simulink model "ospfb_2x_64kc_8i_16o"  (R2021a)
settings: Solver=VariableStepAuto  StartTime=0.0  StopTime=2304  RelTol=1e-3
undefined symbols (not defined in the file or in its folder): pfb_coeff_gen_calc
libraries: xbsIndex_r4 x37193, casper_library_misc x226, casper_library_delays x184
(part 195 of 313)

== system ospfb_2x_64kc_8i_16o/fft2/fft_direct/butterfly3_4/twiddle/coeff_gen/cosin ==
  theta : Inport
  misci : Inport  Port=2
  Constant4 : xbsIndex_r4/Constant  const=0  gui_display_data_type=Fixed-point  arith_type=Unsigned  n_bits=1  bin_pt=0  preci_type=Single  exp_width=8  frac_width=24  explicit_period=on  period=1  equ=P=C  opselect=C  inp2=PCIN>>17  opr=+  inp1=P  carry=CIN  dbl_ovrd=off  block_type=constant
  Constant5 : xbsIndex_r4/Constant  const=0  gui_display_data_type=Fixed-point  arith_type=Unsigned  n_bits=1  bin_pt=0  preci_type=Single  exp_width=8  frac_width=24  explicit_period=on  period=1  equ=P=C  opselect=C  inp2=PCIN>>17  opr=+  inp1=P  carry=CIN  dbl_ovrd=off  block_type=constant
  Delay : xbsIndex_r4/Delay  rst=off  infoeditControl=Selection of Reset will increase slice count due to use of real FFs and instead of SRLs  en=off  latency=bram_latency  reg_retiming=on  dbl_ovrd=off  block_type=delay
  Delay10 : xbsIndex_r4/Delay  rst=off  infoeditControl=Selection of Reset will increase slice count due to use of real FFs and instead of SRLs  en=off  latency=bram_latency  reg_retiming=on  dbl_ovrd=off  block_type=delay
  Delay8 : xbsIndex_r4/Delay  rst=off  infoeditControl=Selection of Reset will increase slice count due to use of real FFs and instead of SRLs  en=off  latency=bram_latency  reg_retiming=on  dbl_ovrd=off  block_type=delay
  ROM : xbsIndex_r4/ROM  depth=2^(12)  initVector=[31744539665 31743228933 31741918202 31740607470 31739296738 31738248151 31736937419 31735626688 31734315956 31733005224 31731956637 31730645905 31729335174 31728024442 31726713710 31725402979 31724354391 31723043660 31721732928 31720422196 31719111465 31718062877 31716752146 31715441414 31714130682 31712819951 31711509219 31710198488 31709149900 31707839168 31706528437 31705217705 31703906974 31702596242 31701547654 31700236923 31698926191 31697615460 31696304728 31694993996 31693683265 31692634677 31691323946 31690013214 31688702483 31687391751 31686081019 31684770288 31683721700 31682410969 31681100237 31679789505 31678478774 31677168042 31675857311 31674808723 31673497991 31672187260 31670876528 31669565797 31668255065 31666944334 31665633602 31664585014 31663274283 31661963551 31660652820 31659342088 31658031357 31656720625 31655409893 31654361306 31653050574 31651739843 31650429111 31649118379 31647807648 31646496916 31645186185 31643875453 31642564722 31641516134 31640205402 31638894671 31637583939 31636273208 31634962476 31633651745 31632341013 31631030281 31629719550 31628670962 31627360231 31626049499 31624738768 31623428036 31622117305 31620806573 31619495841 31618185110 31616874378 31615563647 31614252915 31613204328 31611893596 31610582864 31609272133 31607961401 31606650670 31605339938 31604029207 31602718475 31601407744 31600097012 31598786280 31597475549 31596164817 31594854086 31593805498 31592494767 31591184035 31589873304 31588562572 31587251840 31585941109 31584630377 31583319646 31582008914 31580698183 31579387451 31578076720 31576765988 31575455256 31574144525 31572833793 31571523062 31570212330 31568901599 31567853011 31566542280 31565231548 31563920817 31562610085 31561299353 31559988622 31558677890 31557367159 31556056427 31554745696 31553434964 31552124233 31550813501 31549502770 31548192038 31546881307 31545570575 31544259843 31542949112 31541638380 31540327649 31539016917 31537706186 31536395454 31535084723 31533773991 31532463260 31531152528 31529841797 31528531065 31527220334 31525909602 31524598870 31523288139 31521977407 31520666676 31519355944 31518045213 31516734481 31515423750 31514113018 31512802287 31511491555 31510180824 31508870092 31507559361 31506248629 31504937898 31503627166 31502316435 31501005703 31499694971 31498384240 31497073508 31495762777 31494452045 31493141314 31491830582 31490519851 31489209119 31487898388 31486587656 31485276925 31483966193 31482655462 31481344730 31480033999 31478723267 31477412536 31475839660 31474528929 31473218197 31471907466 31470596734 31469286003 31467975271 31466664540 31465353808 31464043077 31462732345 31461421614 31460110882 31458800151 31457489419 31456178688 31454867956 31453557225 31452246493 31450935762 31449362886 31448052155 31446741423 31445430692 31444119960 31442809229 31441498497 31440187766 31438877034 31437566303 31436255571 31434944840 31433634108 31432323377 31430750501 31429439770 31428129038 31426818307 31425507575 31424196844 31422886112 31421575381 31420264649 31418953918 31417643186 31416332455 31414759579 31413448848 31412138116 31410827385 31409516653 31408205922 31406895190 31405584459 31404273727 31402962996 31401652264 31400079389 31398768657 31397457926 31396147194 31394836463 31393525731 31392215000 31390904268 31389593537 31388020662 31386709930 31385399199 31384088467 31382777736 31381467004 31380156273 31378845541 31377534810 31375961934 31374651203 31373340471 31372029740 31370719008 31369408277 31368097545 31366786814 31365213938 31363903207 31362592475 31361281744 31359971013 31358660281 31357349550 31355776674 31354465943 31353155211 31351844480 31350533748 31349223017 31347912285 31346339410 31345028678 31343717947 31342407215 31341096484 31339785753 31338475021 31336902146 31335591414 31334280683 31332969951 31331659220 31330348488 31328775613 31327464881 31326154150 31324843419 31323532687 31322221956 31320649080 31319338349 31318027617 31316716886 31315406154 31314095423 31312522547 31311211816 31309901085 ...(+45156 chars)]  distributed_mem=Block RAM  rst=off  init_reg=0  en=off  latency=bram_latency  gui_display_data_type=Fixed-point  arith_type=Unsigned  n_bits=n_bits*2  bin_pt=0  preci_type=Single  exp_width=8  frac_width=24  optimize=Speed  use_rpm=on  dbl_ovrd=off  simulation_type=fast_sim  block_type=sprom
  Terminator : Terminator
  Terminator1 : Terminator
  Terminator4 : Terminator
  add_convert0 : Subsystem
  add_convert1 : Subsystem
  assert : xbsIndex_r4/Assert  assert_type=on  type_source=Explicitly  gui_display_data_type=Fixed-point  arith_type=Unsigned  n_bits=12  bin_pt=0  preci_type=Single  exp_width=8  frac_width=24  assert_rate=off  rate_source=Explicitly  period=1  output_port=on  block_type=assert
  c_to_ri : casper_library_misc/c_to_ri  n_bits=n_bits  bin_pt=bin_pt
      settings: SourceProductName=CASPER DSP Blockset
  invert0 : Subsystem  mask{n_bits=n_bits, bin_pt=bin_pt, neg_latency=neg_latency, mux_latency=mux_latency}
      mask prompts: n_bits "bit width"; bin_pt "binary point"; neg_latency "negate latency"; mux_latency "mux latency"
  invert1 : Subsystem  mask{n_bits=n_bits, bin_pt=bin_pt, neg_latency=neg_latency, mux_latency=mux_latency}
      mask prompts: n_bits "bit width"; bin_pt "binary point"; neg_latency "negate latency"; mux_latency "mux latency"
  cos : Outport
  -sin : Outport  Port=2
  misco : Outport  Port=3
  wires:
  theta/1 -> assert/1
  assert/1 -> add_convert0/1(theta), add_convert1/1(theta)
  Constant4/1 -> add_convert0/2(misci)
  add_convert0/3(misco) -> Terminator/1
  misci/1 -> add_convert1/2(misci)
  add_convert1/3(misco) -> Delay/1
  add_convert0/2(add) -> ROM/1
  add_convert1/2(add) -> Terminator4/1
  ROM/1 -> c_to_ri/1
  add_convert1/1(negate) -> Delay8/1
  add_convert0/1(negate) -> Delay10/1
  Delay10/1 -> invert0/1(negate)
  Constant5/1 -> invert0/3(misci)
  c_to_ri/1 -> invert0/2(in)
  Delay8/1 -> invert1/1(negate)
  c_to_ri/2 -> invert1/2(in)
  invert0/2(misco) -> Terminator1/1
  Delay/1 -> invert1/3(misci)
  invert0/1(out) -> cos/1
  invert1/1(out) -> -sin/1
  invert1/2(misco) -> misco/1
